simulink model "large_model"  (Simulink 8.0)
settings: Solver=ode45  StartTime=0.0  StopTime=10.0  RelTol=1e-3
(part 8 of 21)

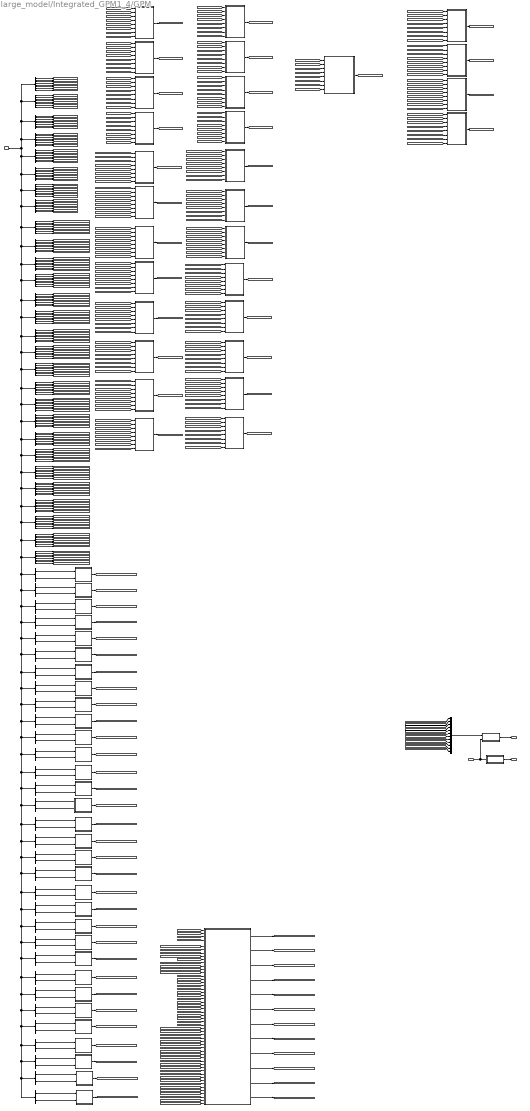
== system large_model/Integrated_GPM1_4/GPM ==
  note: Este componente foi colocado devido a uma limitação do EFTG. Na versão 1.2.1,
      | não é permitido fazer uma conexão direta entre a entrada e a saída.
  From_ES : Inport
  PWR_In : Inport  Port=2
  CAB1_FCC1 : Subsystem
  CAB1_GPM2_fcc_ram_mon_rudder_UPR_dat : BusSelector  OutputSignals=switch2A.switch1A_to_switch.cab1_gpm2_chB.CAB1_GPM2_fcc_ram_mon_rudder_UPR_dat,switch2B.switch1B_to_switch.cab1_gpm2_chB.CAB1_GPM2_fcc_ram_mon_rudder_UPR_dat
  CAB4_GPM2_fcc_ram_mon_AIL_LIB_dat : BusSelector  OutputSignals=switch2A.cab4_gpm2_chA.CAB4_GPM2_fcc_ram_mon_AIL_LIB_dat,switch2B.cab4_gpm2_chB.CAB4_GPM2_fcc_ram_mon_AIL_LIB_dat
  CAB4_GPM2_fcc_ram_mon_AIL_LOB_dat : BusSelector  OutputSignals=switch2A.cab4_gpm2_chA.CAB4_GPM2_fcc_ram_mon_AIL_LOB_dat,switch2B.cab4_gpm2_chB.CAB4_GPM2_fcc_ram_mon_AIL_LOB_dat
  CAB4_GPM2_fcc_ram_mon_AIL_RIB_dat : BusSelector  OutputSignals=switch2A.cab4_gpm2_chA.CAB4_GPM2_fcc_ram_mon_AIL_RIB_dat,switch2B.cab4_gpm2_chB.CAB4_GPM2_fcc_ram_mon_AIL_RIB_dat
  CAB4_GPM2_fcc_ram_mon_AIL_ROB_dat : BusSelector  OutputSignals=switch2A.cab4_gpm2_chA.CAB4_GPM2_fcc_ram_mon_AIL_ROB_dat,switch2B.cab4_gpm2_chB.CAB4_GPM2_fcc_ram_mon_AIL_ROB_dat
  CAB4_GPM2_fcc_ram_mon_ELEV_LIB_dat : BusSelector  OutputSignals=switch2A.cab4_gpm2_chA.CAB4_GPM2_fcc_ram_mon_ELEV_LIB_dat,switch2B.cab4_gpm2_chB.CAB4_GPM2_fcc_ram_mon_ELEV_LIB_dat
  CAB4_GPM2_fcc_ram_mon_ELEV_LOB_dat : BusSelector  OutputSignals=switch2A.cab4_gpm2_chA.CAB4_GPM2_fcc_ram_mon_ELEV_LOB_dat,switch2B.cab4_gpm2_chB.CAB4_GPM2_fcc_ram_mon_ELEV_LOB_dat
  CAB4_GPM2_fcc_ram_mon_ELEV_RIB_dat : BusSelector  OutputSignals=switch2A.cab4_gpm2_chA.CAB4_GPM2_fcc_ram_mon_ELEV_RIB_dat,switch2B.cab4_gpm2_chB.CAB4_GPM2_fcc_ram_mon_ELEV_RIB_dat
  CAB4_GPM2_fcc_ram_mon_ELEV_ROB_dat : BusSelector  OutputSignals=switch2A.cab4_gpm2_chA.CAB4_GPM2_fcc_ram_mon_ELEV_ROB_dat,switch2B.cab4_gpm2_chB.CAB4_GPM2_fcc_ram_mon_ELEV_ROB_dat
  CAB4_GPM2_fcc_ram_mon_rudder_LWR_dat : BusSelector  OutputSignals=switch2A.cab4_gpm2_chA.CAB4_GPM2_fcc_ram_mon_rudder_LWR_dat,switch2B.cab4_gpm2_chB.CAB4_GPM2_fcc_ram_mon_rudder_LWR_dat
  CAB4_GPM2_pitch_roll_cmd : BusSelector  OutputSignals=switch2A.cab4_gpm2_chA.CAB4_GPM2_pitch_roll_cmd,switch2B.cab4_gpm2_chB.CAB4_GPM2_pitch_roll_cmd
  CAB4_GPM2_yaw_cmd : BusSelector  OutputSignals=switch2A.cab4_gpm2_chA.CAB4_GPM2_yaw_cmd,switch2B.cab4_gpm2_chB.CAB4_GPM2_yaw_cmd
  GPM Hardware : Subsystem
  Outputs : BusCreator  Inputs=12
  PWR : Subsystem
  SCP_CAB1_GPM2_fcc_ram_mon_AIL_LIB : Subsystem
  SCP_CAB1_GPM2_fcc_ram_mon_AIL_LOB : Subsystem
  SCP_CAB1_GPM2_fcc_ram_mon_AIL_RIB : Subsystem
  SCP_CAB1_GPM2_fcc_ram_mon_AIL_ROB : Subsystem
  SCP_CAB1_GPM2_fcc_ram_mon_ELEV_LIB : Subsystem
  SCP_CAB1_GPM2_fcc_ram_mon_ELEV_LOB : Subsystem
  SCP_CAB1_GPM2_fcc_ram_mon_ELEV_RIB : Subsystem
  SCP_CAB1_GPM2_fcc_ram_mon_ELEV_ROB : Subsystem
  SCP_CAB1_GPM2_fcc_ram_mon_rudder_LWR : Subsystem
  SCP_CAB1_GPM2_fcc_ram_mon_rudder_UPR : Subsystem
  SCP_CAB1_GPM2_pitch_roll : Subsystem
  SCP_CAB1_GPM2_yaw : Subsystem
  SCP_ail_REU_LIB_LVDT : Subsystem
  SCP_ail_REU_LIB_mon : Subsystem
  SCP_ail_REU_LOB_LVDT : Subsystem
  SCP_ail_REU_LOB_mon : Subsystem
  SCP_ail_REU_RIB_LVDT : Subsystem
  SCP_ail_REU_RIB_mon : Subsystem
  SCP_ail_REU_ROB_LVDT : Subsystem
  SCP_ail_REU_ROB_mon : Subsystem
  SCP_elev_REU_LIB_LVDT : Subsystem
  SCP_elev_REU_LIB_mon_dat : Subsystem
  SCP_elev_REU_LOB_LVDT : Subsystem
  SCP_elev_REU_LOB_mon : Subsystem
  SCP_elev_REU_RIB_LVDT : Subsystem
  SCP_elev_REU_RIB_mon : Subsystem
  SCP_elev_REU_ROB_LVDT : Subsystem
  SCP_elev_REU_ROB_mon : Subsystem
  SCP_rud_REU1_LVDT : Subsystem
  SCP_rud_REU1_MON : Subsystem
  SCP_rud_REU2_LVDT : Subsystem
  SCP_rud_REU2_MON : Subsystem
  Self_checking_pair_ADS1A : Subsystem
  Self_checking_pair_ADS1B : Subsystem
  Self_checking_pair_ADS2A : Subsystem
  Self_checking_pair_ADS2B : Subsystem
  Self_checking_pair_ADS3A : Subsystem
  Self_checking_pair_ADS3B : Subsystem
  Self_checking_pair_ADS4A : Subsystem
  Self_checking_pair_ADS4B : Subsystem
  Self_checking_pair_PedalYaw1 : Subsystem
  Self_checking_pair_PedalYaw2 : Subsystem
  Self_checking_pair_PedalYaw3 : Subsystem
  Self_checking_pair_PedalYaw4 : Subsystem
  Self_checking_pair_SSC1_pitch1 : Subsystem
  Self_checking_pair_SSC1_pitch2 : Subsystem
  Self_checking_pair_SSC1_pitch3 : Subsystem
  Self_checking_pair_SSC1_pitch4 : Subsystem
  Self_checking_pair_SSC1_roll1 : Subsystem
  Self_checking_pair_SSC1_roll2 : Subsystem
  Self_checking_pair_SSC1_roll3 : Subsystem
  Self_checking_pair_SSC1_roll4 : Subsystem
  Self_checking_pair_SSC2_pitch1 : Subsystem
  Self_checking_pair_SSC2_pitch2 : Subsystem
  Self_checking_pair_SSC2_pitch3 : Subsystem
  Self_checking_pair_SSC2_pitch4 : Subsystem
  Self_checking_pair_SSC2_roll1 : Subsystem
  Self_checking_pair_SSC2_roll2 : Subsystem
  Self_checking_pair_SSC2_roll3 : Subsystem
  Self_checking_pair_SSC2_roll4 : Subsystem
  Voter_ADS : Subsystem
  ads_1A : BusSelector  Description=|  OutputSignals=switch2A.cab3_gpm_IO_chA.ads_1A,switch2A.cab4_gpm_IO_chA.ads_1A,switch2A.switch1A_to_switch.cab2_gpm_IO_chB.ads_1A,switch2A.switch1A_to_switch.cab1_gpm_IO_chB.ads_1A,switch2B.cab4_gpm_IO_chB.ads_1A,switch2B.cab3_gpm_IO_chB.ads_1A,switch2B.switch1B_to_switch.cab2_gpm_IO_chB.ads_1A,switch2B.switch1B_to_switch.cab1_gpm_IO_chB.ads_1A
      | When connected to a line which contains bus signals, this bus selector will select as its outputs all the signals which contain this bus selector name.
      | For example, if you name this bus selector as "sidestick_1_pitch_1", all signals containing this string will be selected as its outputs.
  ads_1B : BusSelector  Description=|  OutputSignals=switch2A.cab3_gpm_IO_chA.ads_1B,switch2A.cab4_gpm_IO_chA.ads_1B,switch2A.switch1A_to_switch.cab2_gpm_IO_chB.ads_1B,switch2A.switch1A_to_switch.cab1_gpm_IO_chB.ads_1B,switch2B.cab4_gpm_IO_chB.ads_1B,switch2B.cab3_gpm_IO_chB.ads_1B,switch2B.switch1B_to_switch.cab2_gpm_IO_chB.ads_1B,switch2B.switch1B_to_switch.cab1_gpm_IO_chB.ads_1B
      | When connected to a line which contains bus signals, this bus selector will select as its outputs all the signals which contain this bus selector name.
      | For example, if you name this bus selector as "sidestick_1_pitch_1", all signals containing this string will be selected as its outputs.
  ads_2A : BusSelector  Description=|  OutputSignals=switch2A.cab3_gpm_IO_chA.ads_2A,switch2A.cab4_gpm_IO_chA.ads_2A,switch2A.switch1A_to_switch.cab2_gpm_IO_chB.ads_2A,switch2A.switch1A_to_switch.cab1_gpm_IO_chB.ads_2A,switch2B.cab4_gpm_IO_chB.ads_2A,switch2B.cab3_gpm_IO_chB.ads_2A,switch2B.switch1B_to_switch.cab2_gpm_IO_chB.ads_2A,switch2B.switch1B_to_switch.cab1_gpm_IO_chB.ads_2A
      | When connected to a line which contains bus signals, this bus selector will select as its outputs all the signals which contain this bus selector name.
      | For example, if you name this bus selector as "sidestick_1_pitch_1", all signals containing this string will be selected as its outputs.
  ads_2B : BusSelector  Description=|  OutputSignals=switch2A.cab3_gpm_IO_chA.ads_2B,switch2A.cab4_gpm_IO_chA.ads_2B,switch2A.switch1A_to_switch.cab2_gpm_IO_chB.ads_2B,switch2A.switch1A_to_switch.cab1_gpm_IO_chB.ads_2B,switch2B.cab4_gpm_IO_chB.ads_2B,switch2B.cab3_gpm_IO_chB.ads_2B,switch2B.switch1B_to_switch.cab2_gpm_IO_chB.ads_2B,switch2B.switch1B_to_switch.cab1_gpm_IO_chB.ads_2B
      | When connected to a line which contains bus signals, this bus selector will select as its outputs all the signals which contain this bus selector name.
      | For example, if you name this bus selector as "sidestick_1_pitch_1", all signals containing this string will be selected as its outputs.
  ads_3A : BusSelector  Description=|  OutputSignals=switch2A.cab3_gpm_IO_chA.ads_3A,switch2A.cab4_gpm_IO_chA.ads_3A,switch2A.switch1A_to_switch.cab2_gpm_IO_chB.ads_3A,switch2A.switch1A_to_switch.cab1_gpm_IO_chB.ads_3A,switch2B.cab4_gpm_IO_chB.ads_3A,switch2B.cab3_gpm_IO_chB.ads_3A,switch2B.switch1B_to_switch.cab2_gpm_IO_chB.ads_3A,switch2B.switch1B_to_switch.cab1_gpm_IO_chB.ads_3A
      | When connected to a line which contains bus signals, this bus selector will select as its outputs all the signals which contain this bus selector name.
      | For example, if you name this bus selector as "sidestick_1_pitch_1", all signals containing this string will be selected as its outputs.
  ads_3B : BusSelector  Description=|  OutputSignals=switch2A.cab3_gpm_IO_chA.ads_3B,switch2A.cab4_gpm_IO_chA.ads_3B,switch2A.switch1A_to_switch.cab2_gpm_IO_chB.ads_3B,switch2A.switch1A_to_switch.cab1_gpm_IO_chB.ads_3B,switch2B.cab4_gpm_IO_chB.ads_3B,switch2B.cab3_gpm_IO_chB.ads_3B,switch2B.switch1B_to_switch.cab2_gpm_IO_chB.ads_3B,switch2B.switch1B_to_switch.cab1_gpm_IO_chB.ads_3B
      | When connected to a line which contains bus signals, this bus selector will select as its outputs all the signals which contain this bus selector name.
      | For example, if you name this bus selector as "sidestick_1_pitch_1", all signals containing this string will be selected as its outputs.
  ads_4A : BusSelector  Description=|  OutputSignals=switch2A.cab3_gpm_IO_chA.ads_4A,switch2A.cab4_gpm_IO_chA.ads_4A,switch2A.switch1A_to_switch.cab2_gpm_IO_chB.ads_4A,switch2A.switch1A_to_switch.cab1_gpm_IO_chB.ads_4A,switch2B.cab4_gpm_IO_chB.ads_4A,switch2B.cab3_gpm_IO_chB.ads_4A,switch2B.switch1B_to_switch.cab2_gpm_IO_chB.ads_4A,switch2B.switch1B_to_switch.cab1_gpm_IO_chB.ads_4A
      | When connected to a line which contains bus signals, this bus selector will select as its outputs all the signals which contain this bus selector name.
      | For example, if you name this bus selector as "sidestick_1_pitch_1", all signals containing this string will be selected as its outputs.
  ads_4B : BusSelector  Description=|  OutputSignals=switch2A.cab3_gpm_IO_chA.ads_4B,switch2A.cab4_gpm_IO_chA.ads_4B,switch2A.switch1A_to_switch.cab2_gpm_IO_chB.ads_4B,switch2A.switch1A_to_switch.cab1_gpm_IO_chB.ads_4B,switch2B.cab4_gpm_IO_chB.ads_4B,switch2B.cab3_gpm_IO_chB.ads_4B,switch2B.switch1B_to_switch.cab2_gpm_IO_chB.ads_4B,switch2B.switch1B_to_switch.cab1_gpm_IO_chB.ads_4B
      | When connected to a line which contains bus signals, this bus selector will select as its outputs all the signals which contain this bus selector name.
      | For example, if you name this bus selector as "sidestick_1_pitch_1", all signals containing this string will be selected as its outputs.
  ail_REU_LIB_LVDT_dat : BusSelector  OutputSignals=switch2A.REU_to_Switch2A.ail_REU_LIB_LVDT_dat,switch2B.REU_to_Switch2B.ail_REU_LIB_LVDT_dat
  ail_REU_LIB_mon_dat : BusSelector  OutputSignals=switch2A.REU_to_Switch2A.ail_REU_LIB_mon_dat,switch2B.REU_to_Switch2B.ail_REU_LIB_mon_dat
  ail_REU_LOB_LVDT_dat : BusSelector  OutputSignals=switch2A.switch1A_to_switch.REU_to_Switch1A.ail_REU_LOB_LVDT_dat,switch2B.switch1B_to_switch.REU_to_Switch1B.ail_REU_LOB_LVDT_dat
  ail_REU_LOB_mon_dat : BusSelector  OutputSignals=switch2A.switch1A_to_switch.REU_to_Switch1A.ail_REU_LOB_mon_dat,switch2B.switch1B_to_switch.REU_to_Switch1B.ail_REU_LOB_mon_dat
  ail_REU_RIB_LVDT_dat : BusSelector  OutputSignals=switch2A.REU_to_Switch2A.ail_REU_RIB_LVDT_dat,switch2B.REU_to_Switch2B.ail_REU_RIB_LVDT_dat
  ail_REU_RIB_mon_dat : BusSelector  OutputSignals=switch2A.REU_to_Switch2A.ail_REU_RIB_mon_dat,switch2B.REU_to_Switch2B.ail_REU_RIB_mon_dat
  ail_REU_ROB_LVDT_dat : BusSelector  OutputSignals=switch2A.switch1A_to_switch.REU_to_Switch1A.ail_REU_ROB_LVDT_dat,switch2B.switch1B_to_switch.REU_to_Switch1B.ail_REU_ROB_LVDT_dat
  ail_REU_ROB_mon_dat : BusSelector  OutputSignals=switch2A.switch1A_to_switch.REU_to_Switch1A.ail_REU_ROB_mon_dat,switch2B.switch1B_to_switch.REU_to_Switch1B.ail_REU_ROB_mon_dat
  elev_REU_LIB_LVDT_dat : BusSelector  OutputSignals=switch2A.REU_to_Switch2A.elev_REU_LIB_LVDT_dat,switch2B.REU_to_Switch2B.elev_REU_LIB_LVDT_dat
  elev_REU_LIB_mon_dat : BusSelector  OutputSignals=switch2A.REU_to_Switch2A.elev_REU_LIB_mon_dat,switch2B.REU_to_Switch2B.elev_REU_LIB_mon_dat
  elev_REU_LOB_LVDT_dat : BusSelector  OutputSignals=switch2A.switch1A_to_switch.REU_to_Switch1A.elev_REU_LOB_LVDT_dat,switch2B.switch1B_to_switch.REU_to_Switch1B.elev_REU_LOB_LVDT_dat
  elev_REU_LOB_mon_dat : BusSelector  OutputSignals=switch2A.switch1A_to_switch.REU_to_Switch1A.elev_REU_LOB_mon_dat,switch2B.switch1B_to_switch.REU_to_Switch1B.elev_REU_LOB_mon_dat
  elev_REU_RIB_LVDT_dat : BusSelector  OutputSignals=switch2A.REU_to_Switch2A.elev_REU_RIB_LVDT_dat,switch2B.REU_to_Switch2B.elev_REU_RIB_LVDT_dat
  elev_REU_RIB_mon_dat : BusSelector  OutputSignals=switch2A.REU_to_Switch2A.elev_REU_RIB_mon_dat,switch2B.REU_to_Switch2B.elev_REU_RIB_mon_dat
  elev_REU_ROB_LVDT_dat : BusSelector  OutputSignals=switch2A.switch1A_to_switch.REU_to_Switch1A.elev_REU_ROB_LVDT_dat,switch2B.switch1B_to_switch.REU_to_Switch1B.elev_REU_ROB_LVDT_dat
  elev_REU_ROB_mon_dat : BusSelector  OutputSignals=switch2A.switch1A_to_switch.REU_to_Switch1A.elev_REU_ROB_mon_dat,switch2B.switch1B_to_switch.REU_to_Switch1B.elev_REU_ROB_mon_dat
  pedal_yaw_1 : BusSelector  Description=|  OutputSignals=switch2A.cab3_gpm_IO_chA.rdc_4002_1.pedal_yaw_1,switch2A.cab4_gpm_IO_chA.rdc_4002_1.pedal_yaw_1,switch2A.switch1A_to_switch.cab2_gpm_IO_chB.rdc_4002_1.pedal_yaw_1,switch2A.switch1A_to_switch.cab1_gpm_IO_chB.rdc_4002_1.pedal_yaw_1,switch2B.cab4_gpm_IO_chB.rdc_4002_1.pedal_yaw_1,switch2B.cab3_gpm_IO_chB.rdc_4002_1.pedal_yaw_1,switch2B.switch1B_to_switch.cab2_gpm_IO_chB.rdc_4002_1.pedal_yaw_1,switch2B.switch1B_to_switch.cab1_gpm_IO_chB.rdc_4002_1.pedal_yaw_1
      | When connected to a line which contains bus signals, this bus selector will select as its outputs all the signals which contain this bus selector name.
      | For example, if you name this bus selector as "sidestick_1_pitch_1", all signals containing this string will be selected as its outputs.
  pedal_yaw_2 : BusSelector  Description=|  OutputSignals=switch2A.cab3_gpm_IO_chA.rdc_4002_2.pedal_yaw_2,switch2A.cab4_gpm_IO_chA.rdc_4002_2.pedal_yaw_2,switch2A.switch1A_to_switch.cab2_gpm_IO_chB.rdc_4002_2.pedal_yaw_2,switch2A.switch1A_to_switch.cab1_gpm_IO_chB.rdc_4002_2.pedal_yaw_2,switch2B.cab4_gpm_IO_chB.rdc_4002_2.pedal_yaw_2,switch2B.cab3_gpm_IO_chB.rdc_4002_2.pedal_yaw_2,switch2B.switch1B_to_switch.cab2_gpm_IO_chB.rdc_4002_2.pedal_yaw_2,switch2B.switch1B_to_switch.cab1_gpm_IO_chB.rdc_4002_2.pedal_yaw_2
      | When connected to a line which contains bus signals, this bus selector will select as its outputs all the signals which contain this bus selector name.
      | For example, if you name this bus selector as "sidestick_1_pitch_1", all signals containing this string will be selected as its outputs.
  pedal_yaw_3 : BusSelector  Description=|  OutputSignals=switch2A.cab3_gpm_IO_chA.rdc_4002_3.pedal_yaw_3,switch2A.cab4_gpm_IO_chA.rdc_4002_3.pedal_yaw_3,switch2A.switch1A_to_switch.cab2_gpm_IO_chB.rdc_4002_3.pedal_yaw_3,switch2A.switch1A_to_switch.cab1_gpm_IO_chB.rdc_4002_3.pedal_yaw_3,switch2B.cab4_gpm_IO_chB.rdc_4002_3.pedal_yaw_3,switch2B.cab3_gpm_IO_chB.rdc_4002_3.pedal_yaw_3,switch2B.switch1B_to_switch.cab2_gpm_IO_chB.rdc_4002_3.pedal_yaw_3,switch2B.switch1B_to_switch.cab1_gpm_IO_chB.rdc_4002_3.pedal_yaw_3
      | When connected to a line which contains bus signals, this bus selector will select as its outputs all the signals which contain this bus selector name.
      | For example, if you name this bus selector as "sidestick_1_pitch_1", all signals containing this string will be selected as its outputs.
  pedal_yaw_4 : BusSelector  Description=|  OutputSignals=switch2A.cab3_gpm_IO_chA.rdc_4002_4.pedal_yaw_4,switch2A.cab4_gpm_IO_chA.rdc_4002_4.pedal_yaw_4,switch2A.switch1A_to_switch.cab2_gpm_IO_chB.rdc_4002_4.pedal_yaw_4,switch2A.switch1A_to_switch.cab1_gpm_IO_chB.rdc_4002_4.pedal_yaw_4,switch2B.cab4_gpm_IO_chB.rdc_4002_4.pedal_yaw_4,switch2B.cab3_gpm_IO_chB.rdc_4002_4.pedal_yaw_4,switch2B.switch1B_to_switch.cab2_gpm_IO_chB.rdc_4002_4.pedal_yaw_4,switch2B.switch1B_to_switch.cab1_gpm_IO_chB.rdc_4002_4.pedal_yaw_4
      | When connected to a line which contains bus signals, this bus selector will select as its outputs all the signals which contain this bus selector name.
      | For example, if you name this bus selector as "sidestick_1_pitch_1", all signals containing this string will be selected as its outputs.
  rud_REU1_LVDT_dat : BusSelector  OutputSignals=switch2A.REU_to_Switch2A.rud_REU1_LVDT_dat,switch2B.REU_to_Switch2B.rud_REU1_LVDT_dat
  rud_REU1_MON_dat : BusSelector  OutputSignals=switch2A.REU_to_Switch2A.rud_REU1_MON_dat,switch2B.REU_to_Switch2B.rud_REU1_MON_dat
  rud_REU2_LVDT_dat : BusSelector  OutputSignals=switch2A.switch1A_to_switch.REU_to_Switch1A.rud_REU2_LVDT_dat,switch2B.switch1B_to_switch.REU_to_Switch1B.rud_REU2_LVDT_dat
  rud_REU2_MON_dat : BusSelector  OutputSignals=switch2A.switch1A_to_switch.REU_to_Switch1A.rud_REU2_MON_dat,switch2B.switch1B_to_switch.REU_to_Switch1B.rud_REU2_MON_dat
  sidestick1_pitch_1 : BusSelector  OutputSignals=switch2A.cab3_gpm_IO_chA.rdc_4002_1.sidesticks.sidestick1_pitch_1,switch2A.cab4_gpm_IO_chA.rdc_4002_1.sidesticks.sidestick1_pitch_1,switch2A.switch1A_to_switch.cab2_gpm_IO_chB.rdc_4002_1.sidesticks.sidestick1_pitch_1,switch2A.switch1A_to_switch.cab1_gpm_IO_chB.rdc_4002_1.sidesticks.sidestick1_pitch_1,switch2B.cab4_gpm_IO_chB.rdc_4002_1.sidesticks.sidestick1_pitch_1,switch2B.cab3_gpm_IO_chB.rdc_4002_1.sidesticks.sidestick1_pitch_1,switch2B.switch1B_to_switch.cab2_gpm_IO_chB.rdc_4002_1.sidesticks.sidestick1_pitch_1,switch2B.switch1B_to_switch.cab1_gpm_IO_chB.rdc_4002_1.sidesticks.sidestick1_pitch_1
  sidestick1_pitch_2 : BusSelector  OutputSignals=switch2A.cab3_gpm_IO_chA.rdc_4002_2.sidesticks.sidestick1_pitch_2,switch2A.cab4_gpm_IO_chA.rdc_4002_2.sidesticks.sidestick1_pitch_2,switch2A.switch1A_to_switch.cab2_gpm_IO_chB.rdc_4002_2.sidesticks.sidestick1_pitch_2,switch2A.switch1A_to_switch.cab1_gpm_IO_chB.rdc_4002_2.sidesticks.sidestick1_pitch_2,switch2B.cab4_gpm_IO_chB.rdc_4002_2.sidesticks.sidestick1_pitch_2,switch2B.cab3_gpm_IO_chB.rdc_4002_2.sidesticks.sidestick1_pitch_2,switch2B.switch1B_to_switch.cab2_gpm_IO_chB.rdc_4002_2.sidesticks.sidestick1_pitch_2,switch2B.switch1B_to_switch.cab1_gpm_IO_chB.rdc_4002_2.sidesticks.sidestick1_pitch_2
  sidestick1_pitch_3 : BusSelector  OutputSignals=switch2A.cab3_gpm_IO_chA.rdc_4002_3.sidesticks.sidestick1_pitch_3,switch2A.cab4_gpm_IO_chA.rdc_4002_3.sidesticks.sidestick1_pitch_3,switch2A.switch1A_to_switch.cab2_gpm_IO_chB.rdc_4002_3.sidesticks.sidestick1_pitch_3,switch2A.switch1A_to_switch.cab1_gpm_IO_chB.rdc_4002_3.sidesticks.sidestick1_pitch_3,switch2B.cab4_gpm_IO_chB.rdc_4002_3.sidesticks.sidestick1_pitch_3,switch2B.cab3_gpm_IO_chB.rdc_4002_3.sidesticks.sidestick1_pitch_3,switch2B.switch1B_to_switch.cab2_gpm_IO_chB.rdc_4002_3.sidesticks.sidestick1_pitch_3,switch2B.switch1B_to_switch.cab1_gpm_IO_chB.rdc_4002_3.sidesticks.sidestick1_pitch_3
  sidestick1_pitch_4 : BusSelector  OutputSignals=switch2A.cab3_gpm_IO_chA.rdc_4002_4.sidesticks.sidestick1_pitch_4,switch2A.cab4_gpm_IO_chA.rdc_4002_4.sidesticks.sidestick1_pitch_4,switch2A.switch1A_to_switch.cab2_gpm_IO_chB.rdc_4002_4.sidesticks.sidestick1_pitch_4,switch2A.switch1A_to_switch.cab1_gpm_IO_chB.rdc_4002_4.sidesticks.sidestick1_pitch_4,switch2B.cab4_gpm_IO_chB.rdc_4002_4.sidesticks.sidestick1_pitch_4,switch2B.cab3_gpm_IO_chB.rdc_4002_4.sidesticks.sidestick1_pitch_4,switch2B.switch1B_to_switch.cab2_gpm_IO_chB.rdc_4002_4.sidesticks.sidestick1_pitch_4,switch2B.switch1B_to_switch.cab1_gpm_IO_chB.rdc_4002_4.sidesticks.sidestick1_pitch_4
  sidestick1_roll_1 : BusSelector  OutputSignals=switch2A.cab3_gpm_IO_chA.rdc_4002_1.sidesticks.sidestick1_roll_1,switch2A.cab4_gpm_IO_chA.rdc_4002_1.sidesticks.sidestick1_roll_1,switch2A.switch1A_to_switch.cab2_gpm_IO_chB.rdc_4002_1.sidesticks.sidestick1_roll_1,switch2A.switch1A_to_switch.cab1_gpm_IO_chB.rdc_4002_1.sidesticks.sidestick1_roll_1,switch2B.cab4_gpm_IO_chB.rdc_4002_1.sidesticks.sidestick1_roll_1,switch2B.cab3_gpm_IO_chB.rdc_4002_1.sidesticks.sidestick1_roll_1,switch2B.switch1B_to_switch.cab2_gpm_IO_chB.rdc_4002_1.sidesticks.sidestick1_roll_1,switch2B.switch1B_to_switch.cab1_gpm_IO_chB.rdc_4002_1.sidesticks.sidestick1_roll_1
  sidestick1_roll_2 : BusSelector  OutputSignals=switch2A.cab3_gpm_IO_chA.rdc_4002_2.sidesticks.sidestick1_roll_2,switch2A.cab4_gpm_IO_chA.rdc_4002_2.sidesticks.sidestick1_roll_2,switch2A.switch1A_to_switch.cab2_gpm_IO_chB.rdc_4002_2.sidesticks.sidestick1_roll_2,switch2A.switch1A_to_switch.cab1_gpm_IO_chB.rdc_4002_2.sidesticks.sidestick1_roll_2,switch2B.cab4_gpm_IO_chB.rdc_4002_2.sidesticks.sidestick1_roll_2,switch2B.cab3_gpm_IO_chB.rdc_4002_2.sidesticks.sidestick1_roll_2,switch2B.switch1B_to_switch.cab2_gpm_IO_chB.rdc_4002_2.sidesticks.sidestick1_roll_2,switch2B.switch1B_to_switch.cab1_gpm_IO_chB.rdc_4002_2.sidesticks.sidestick1_roll_2
  sidestick1_roll_3 : BusSelector  OutputSignals=switch2A.cab3_gpm_IO_chA.rdc_4002_3.sidesticks.sidestick1_roll_3,switch2A.cab4_gpm_IO_chA.rdc_4002_3.sidesticks.sidestick1_roll_3,switch2A.switch1A_to_switch.cab2_gpm_IO_chB.rdc_4002_3.sidesticks.sidestick1_roll_3,switch2A.switch1A_to_switch.cab1_gpm_IO_chB.rdc_4002_3.sidesticks.sidestick1_roll_3,switch2B.cab4_gpm_IO_chB.rdc_4002_3.sidesticks.sidestick1_roll_3,switch2B.cab3_gpm_IO_chB.rdc_4002_3.sidesticks.sidestick1_roll_3,switch2B.switch1B_to_switch.cab2_gpm_IO_chB.rdc_4002_3.sidesticks.sidestick1_roll_3,switch2B.switch1B_to_switch.cab1_gpm_IO_chB.rdc_4002_3.sidesticks.sidestick1_roll_3
  sidestick1_roll_4 : BusSelector  OutputSignals=switch2A.cab3_gpm_IO_chA.rdc_4002_4.sidesticks.sidestick1_roll_4,switch2A.cab4_gpm_IO_chA.rdc_4002_4.sidesticks.sidestick1_roll_4,switch2A.switch1A_to_switch.cab2_gpm_IO_chB.rdc_4002_4.sidesticks.sidestick1_roll_4,switch2A.switch1A_to_switch.cab1_gpm_IO_chB.rdc_4002_4.sidesticks.sidestick1_roll_4,switch2B.cab4_gpm_IO_chB.rdc_4002_4.sidesticks.sidestick1_roll_4,switch2B.cab3_gpm_IO_chB.rdc_4002_4.sidesticks.sidestick1_roll_4,switch2B.switch1B_to_switch.cab2_gpm_IO_chB.rdc_4002_4.sidesticks.sidestick1_roll_4,switch2B.switch1B_to_switch.cab1_gpm_IO_chB.rdc_4002_4.sidesticks.sidestick1_roll_4
  sidestick2_pitch_1 : BusSelector  OutputSignals=switch2A.cab3_gpm_IO_chA.rdc_4002_1.sidesticks.sidestick2_pitch_1,switch2A.cab4_gpm_IO_chA.rdc_4002_1.sidesticks.sidestick2_pitch_1,switch2A.switch1A_to_switch.cab2_gpm_IO_chB.rdc_4002_1.sidesticks.sidestick2_pitch_1,switch2A.switch1A_to_switch.cab1_gpm_IO_chB.rdc_4002_1.sidesticks.sidestick2_pitch_1,switch2B.cab4_gpm_IO_chB.rdc_4002_1.sidesticks.sidestick2_pitch_1,switch2B.cab3_gpm_IO_chB.rdc_4002_1.sidesticks.sidestick2_pitch_1,switch2B.switch1B_to_switch.cab2_gpm_IO_chB.rdc_4002_1.sidesticks.sidestick2_pitch_1,switch2B.switch1B_to_switch.cab1_gpm_IO_chB.rdc_4002_1.sidesticks.sidestick2_pitch_1
  sidestick2_pitch_2 : BusSelector  OutputSignals=switch2A.cab3_gpm_IO_chA.rdc_4002_2.sidesticks.sidestick2_pitch_2,switch2A.cab4_gpm_IO_chA.rdc_4002_2.sidesticks.sidestick2_pitch_2,switch2A.switch1A_to_switch.cab2_gpm_IO_chB.rdc_4002_2.sidesticks.sidestick2_pitch_2,switch2A.switch1A_to_switch.cab1_gpm_IO_chB.rdc_4002_2.sidesticks.sidestick2_pitch_2,switch2B.cab4_gpm_IO_chB.rdc_4002_2.sidesticks.sidestick2_pitch_2,switch2B.cab3_gpm_IO_chB.rdc_4002_2.sidesticks.sidestick2_pitch_2,switch2B.switch1B_to_switch.cab2_gpm_IO_chB.rdc_4002_2.sidesticks.sidestick2_pitch_2,switch2B.switch1B_to_switch.cab1_gpm_IO_chB.rdc_4002_2.sidesticks.sidestick2_pitch_2
  sidestick2_pitch_3 : BusSelector  OutputSignals=switch2A.cab3_gpm_IO_chA.rdc_4002_3.sidesticks.sidestick2_pitch_3,switch2A.cab4_gpm_IO_chA.rdc_4002_3.sidesticks.sidestick2_pitch_3,switch2A.switch1A_to_switch.cab2_gpm_IO_chB.rdc_4002_3.sidesticks.sidestick2_pitch_3,switch2A.switch1A_to_switch.cab1_gpm_IO_chB.rdc_4002_3.sidesticks.sidestick2_pitch_3,switch2B.cab4_gpm_IO_chB.rdc_4002_3.sidesticks.sidestick2_pitch_3,switch2B.cab3_gpm_IO_chB.rdc_4002_3.sidesticks.sidestick2_pitch_3,switch2B.switch1B_to_switch.cab2_gpm_IO_chB.rdc_4002_3.sidesticks.sidestick2_pitch_3,switch2B.switch1B_to_switch.cab1_gpm_IO_chB.rdc_4002_3.sidesticks.sidestick2_pitch_3
  sidestick2_pitch_4 : BusSelector  OutputSignals=switch2A.cab3_gpm_IO_chA.rdc_4002_4.sidesticks.sidestick2_pitch_4,switch2A.cab4_gpm_IO_chA.rdc_4002_4.sidesticks.sidestick2_pitch_4,switch2A.switch1A_to_switch.cab2_gpm_IO_chB.rdc_4002_4.sidesticks.sidestick2_pitch_4,switch2A.switch1A_to_switch.cab1_gpm_IO_chB.rdc_4002_4.sidesticks.sidestick2_pitch_4,switch2B.cab4_gpm_IO_chB.rdc_4002_4.sidesticks.sidestick2_pitch_4,switch2B.cab3_gpm_IO_chB.rdc_4002_4.sidesticks.sidestick2_pitch_4,switch2B.switch1B_to_switch.cab2_gpm_IO_chB.rdc_4002_4.sidesticks.sidestick2_pitch_4,switch2B.switch1B_to_switch.cab1_gpm_IO_chB.rdc_4002_4.sidesticks.sidestick2_pitch_4
  sidestick2_roll_1 : BusSelector  OutputSignals=switch2A.cab3_gpm_IO_chA.rdc_4002_1.sidesticks.sidestick2_roll_1,switch2A.cab4_gpm_IO_chA.rdc_4002_1.sidesticks.sidestick2_roll_1,switch2A.switch1A_to_switch.cab2_gpm_IO_chB.rdc_4002_1.sidesticks.sidestick2_roll_1,switch2A.switch1A_to_switch.cab1_gpm_IO_chB.rdc_4002_1.sidesticks.sidestick2_roll_1,switch2B.cab4_gpm_IO_chB.rdc_4002_1.sidesticks.sidestick2_roll_1,switch2B.cab3_gpm_IO_chB.rdc_4002_1.sidesticks.sidestick2_roll_1,switch2B.switch1B_to_switch.cab2_gpm_IO_chB.rdc_4002_1.sidesticks.sidestick2_roll_1,switch2B.switch1B_to_switch.cab1_gpm_IO_chB.rdc_4002_1.sidesticks.sidestick2_roll_1
  sidestick2_roll_2 : BusSelector  OutputSignals=switch2A.cab3_gpm_IO_chA.rdc_4002_2.sidesticks.sidestick2_roll_2,switch2A.cab4_gpm_IO_chA.rdc_4002_2.sidesticks.sidestick2_roll_2,switch2A.switch1A_to_switch.cab2_gpm_IO_chB.rdc_4002_2.sidesticks.sidestick2_roll_2,switch2A.switch1A_to_switch.cab1_gpm_IO_chB.rdc_4002_2.sidesticks.sidestick2_roll_2,switch2B.cab4_gpm_IO_chB.rdc_4002_2.sidesticks.sidestick2_roll_2,switch2B.cab3_gpm_IO_chB.rdc_4002_2.sidesticks.sidestick2_roll_2,switch2B.switch1B_to_switch.cab2_gpm_IO_chB.rdc_4002_2.sidesticks.sidestick2_roll_2,switch2B.switch1B_to_switch.cab1_gpm_IO_chB.rdc_4002_2.sidesticks.sidestick2_roll_2
  sidestick2_roll_3 : BusSelector  OutputSignals=switch2A.cab3_gpm_IO_chA.rdc_4002_3.sidesticks.sidestick2_roll_3,switch2A.cab4_gpm_IO_chA.rdc_4002_3.sidesticks.sidestick2_roll_3,switch2A.switch1A_to_switch.cab2_gpm_IO_chB.rdc_4002_3.sidesticks.sidestick2_roll_3,switch2A.switch1A_to_switch.cab1_gpm_IO_chB.rdc_4002_3.sidesticks.sidestick2_roll_3,switch2B.cab4_gpm_IO_chB.rdc_4002_3.sidesticks.sidestick2_roll_3,switch2B.cab3_gpm_IO_chB.rdc_4002_3.sidesticks.sidestick2_roll_3,switch2B.switch1B_to_switch.cab2_gpm_IO_chB.rdc_4002_3.sidesticks.sidestick2_roll_3,switch2B.switch1B_to_switch.cab1_gpm_IO_chB.rdc_4002_3.sidesticks.sidestick2_roll_3
  sidestick2_roll_4 : BusSelector  OutputSignals=switch2A.cab3_gpm_IO_chA.rdc_4002_4.sidesticks.sidestick2_roll_4,switch2A.cab4_gpm_IO_chA.rdc_4002_4.sidesticks.sidestick2_roll_4,switch2A.switch1A_to_switch.cab2_gpm_IO_chB.rdc_4002_4.sidesticks.sidestick2_roll_4,switch2A.switch1A_to_switch.cab1_gpm_IO_chB.rdc_4002_4.sidesticks.sidestick2_roll_4,switch2B.cab4_gpm_IO_chB.rdc_4002_4.sidesticks.sidestick2_roll_4,switch2B.cab3_gpm_IO_chB.rdc_4002_4.sidesticks.sidestick2_roll_4,switch2B.switch1B_to_switch.cab2_gpm_IO_chB.rdc_4002_4.sidesticks.sidestick2_roll_4,switch2B.switch1B_to_switch.cab1_gpm_IO_chB.rdc_4002_4.sidesticks.sidestick2_roll_4
  To_ES : Outport
  PWR_Out : Outport  Port=2
  wires:
  [SCP_CAB1_GPM2_yaw_1] -> CAB1_FCC1/6(yaw_GPM2_cmd)
  SCP_CAB1_GPM2_yaw/1(Out) -> [SCP_CAB1_GPM2_yaw_1]
  [SCP_CAB1_GPM2_fcc_ram_mon_rudder_LWR_1] -> CAB1_FCC1/45(fcc_ram_mon_rudder_LWR_GPM2_dat)
  SCP_CAB1_GPM2_fcc_ram_mon_rudder_LWR/1(Out) -> [SCP_CAB1_GPM2_fcc_ram_mon_rudder_LWR_1]
  [SCP_CAB1_GPM2_fcc_ram_mon_AIL_ROB_1] -> CAB1_FCC1/50(fcc_ram_mon_AIL_ROB_GPM2_dat)
  SCP_CAB1_GPM2_fcc_ram_mon_AIL_ROB/1(Out) -> [SCP_CAB1_GPM2_fcc_ram_mon_AIL_ROB_1]
  [SCP_CAB1_GPM2_fcc_ram_mon_AIL_RIB_1] -> CAB1_FCC1/49(fcc_ram_mon_AIL_RIB_GPM2_dat)
  SCP_CAB1_GPM2_fcc_ram_mon_AIL_RIB/1(Out) -> [SCP_CAB1_GPM2_fcc_ram_mon_AIL_RIB_1]
  [SCP_CAB1_GPM2_fcc_ram_mon_AIL_LOB_1] -> CAB1_FCC1/48(fcc_ram_mon_AIL_LOB_GPM2_dat)
  SCP_CAB1_GPM2_fcc_ram_mon_AIL_LOB/1(Out) -> [SCP_CAB1_GPM2_fcc_ram_mon_AIL_LOB_1]
  [SCP_CAB1_GPM2_fcc_ram_mon_AIL_LIB_1] -> CAB1_FCC1/47(fcc_ram_mon_AIL_LIB_GPM2_dat)
  SCP_CAB1_GPM2_fcc_ram_mon_AIL_LIB/1(Out) -> [SCP_CAB1_GPM2_fcc_ram_mon_AIL_LIB_1]
  [SCP_CAB1_GPM2_fcc_ram_mon_rudder_UPR_1] -> CAB1_FCC1/46(fcc_ram_mon_rudder_UPR_GPM2_dat)
  SCP_CAB1_GPM2_fcc_ram_mon_rudder_UPR/1(Out) -> [SCP_CAB1_GPM2_fcc_ram_mon_rudder_UPR_1]
  [SCP_CAB1_GPM2_pitch_roll_1] -> CAB1_FCC1/31(pitch_roll_GPM2_cmd)
  SCP_CAB1_GPM2_pitch_roll/1(Out) -> [SCP_CAB1_GPM2_pitch_roll_1]
  [SCP_CAB1_GPM2_fcc_ram_mon_ELEV_ROB_1] -> CAB1_FCC1/54(fcc_ram_mon_ELEV_ROB_GPM2_dat)
  SCP_CAB1_GPM2_fcc_ram_mon_ELEV_ROB/1(Out) -> [SCP_CAB1_GPM2_fcc_ram_mon_ELEV_ROB_1]
  [SCP_CAB1_GPM2_fcc_ram_mon_ELEV_RIB_1] -> CAB1_FCC1/53(fcc_ram_mon_ELEV_RIB_GPM2_da)
  SCP_CAB1_GPM2_fcc_ram_mon_ELEV_RIB/1(Out) -> [SCP_CAB1_GPM2_fcc_ram_mon_ELEV_RIB_1]
  [SCP_CAB1_GPM2_fcc_ram_mon_ELEV_LOB_1] -> CAB1_FCC1/52(fcc_ram_mon_ELEV_LOB_GPM2_dat)
  SCP_CAB1_GPM2_fcc_ram_mon_ELEV_LOB/1(Out) -> [SCP_CAB1_GPM2_fcc_ram_mon_ELEV_LOB_1]
  [SCP_CAB1_GPM2_fcc_ram_mon_ELEV_LIB_1] -> CAB1_FCC1/51(fcc_ram_mon_ELEV_LIB_GPM2_dat)
  SCP_CAB1_GPM2_fcc_ram_mon_ELEV_LIB/1(Out) -> [SCP_CAB1_GPM2_fcc_ram_mon_ELEV_LIB_1]
  [SCP_elev_REU_ROB_mon_1] -> CAB1_FCC1/44(REU_ELEV_mon_ROB_dat)
  SCP_elev_REU_ROB_mon/1(Out) -> [SCP_elev_REU_ROB_mon_1]
  [SCP_ail_REU_LIB_mon_1] -> CAB1_FCC1/8(REU_mon_AIL_LIB_dat)
  SCP_ail_REU_LIB_mon/1(Out) -> [SCP_ail_REU_LIB_mon_1]
  [SCP_ail_REU_LOB_LVDT_1] -> CAB1_FCC1/9(REU_LVDT_AIL_LOB_dat)
  SCP_ail_REU_LOB_LVDT/1(Out) -> [SCP_ail_REU_LOB_LVDT_1]
  [SCP_ail_REU_RIB_LVDT_1] -> CAB1_FCC1/11(REU_AIL_LVDT_RIB_dat)
  SCP_ail_REU_RIB_LVDT/1(Out) -> [SCP_ail_REU_RIB_LVDT_1]
  [SCP_ail_REU_RIB_mon_1] -> CAB1_FCC1/12(REU_AIL_mon_RIB_dat)
  SCP_ail_REU_RIB_mon/1(Out) -> [SCP_ail_REU_RIB_mon_1]
  [SCP_ail_REU_ROB_LVDT_1] -> CAB1_FCC1/13(REU_AIL_LVDT_ROB_dat)
  SCP_ail_REU_ROB_LVDT/1(Out) -> [SCP_ail_REU_ROB_LVDT_1]
  [SCP_ail_REU_ROB_mon_1] -> CAB1_FCC1/14(REU_AIL_mon_ROB_dat)
  SCP_ail_REU_ROB_mon/1(Out) -> [SCP_ail_REU_ROB_mon_1]
  [SCP_elev_REU_LIB_LVDT_1] -> CAB1_FCC1/32(REU_ELEV_LVDT_LIB_dat)
  SCP_elev_REU_LIB_LVDT/1(Out) -> [SCP_elev_REU_LIB_LVDT_1]
  [SCP_elev_REU_LIB_mon_dat_1] -> CAB1_FCC1/33(REU_ELEV_mon_LIB_dat)
  SCP_elev_REU_LIB_mon_dat/1(Out) -> [SCP_elev_REU_LIB_mon_dat_1]
  [SCP_elev_REU_LOB_LVDT_1] -> CAB1_FCC1/34(REU_ELEV_LVDT_LOB_dat)
  SCP_elev_REU_LOB_LVDT/1(Out) -> [SCP_elev_REU_LOB_LVDT_1]
  [SCP_elev_REU_LOB_mon_1] -> CAB1_FCC1/35(REU_ELEV_mon_LOB_dat)
  SCP_elev_REU_LOB_mon/1(Out) -> [SCP_elev_REU_LOB_mon_1]
  [SCP_rud_REU1_LVDT_1] -> CAB1_FCC1/36(REU1_LVDT_rudder_dat)
  SCP_rud_REU1_LVDT/1(Out) -> [SCP_rud_REU1_LVDT_1]
  [SCP_rud_REU1_MON_1] -> CAB1_FCC1/37(REU1_MON_rudder_dat)
  SCP_rud_REU1_MON/1(Out) -> [SCP_rud_REU1_MON_1]
  [SCP_rud_REU2_LVDT_1] -> CAB1_FCC1/38(REU2_LVDT_rudder_dat)
  SCP_rud_REU2_LVDT/1(Out) -> [SCP_rud_REU2_LVDT_1]
  [SCP_rud_REU2_MON_1] -> CAB1_FCC1/39(REU2_MON_rudder_dat)
  SCP_rud_REU2_MON/1(Out) -> [SCP_rud_REU2_MON_1]
  [SCP_ail_REU_LOB_mon_1] -> CAB1_FCC1/40(REU_mon_AIL_LOB_dat)
  SCP_ail_REU_LOB_mon/1(Out) -> [SCP_ail_REU_LOB_mon_1]
  [SCP_elev_REU_RIB_LVDT_1] -> CAB1_FCC1/41(REU_ELEV_LVDT_RIB_dat)
  SCP_elev_REU_RIB_LVDT/1(Out) -> [SCP_elev_REU_RIB_LVDT_1]
  [SCP_elev_REU_RIB_mon_1] -> CAB1_FCC1/42(REU_ELEV_mon_RIB_dat)
  SCP_elev_REU_RIB_mon/1(Out) -> [SCP_elev_REU_RIB_mon_1]
  [SCP_elev_REU_ROB_LVDT_1] -> CAB1_FCC1/43(REU_ELEV_LVDT_ROB_dat)
  SCP_elev_REU_ROB_LVDT/1(Out) -> [SCP_elev_REU_ROB_LVDT_1]
  [SCP_ail_REU_LIB_LVDT_1] -> CAB1_FCC1/7(REU_LVDT_AIL_LIB_dat)
  SCP_ail_REU_LIB_LVDT/1(Out) -> [SCP_ail_REU_LIB_LVDT_1]
  CAB4_GPM2_fcc_ram_mon_ELEV_ROB_dat/2 -> SCP_CAB1_GPM2_fcc_ram_mon_ELEV_ROB/2(In2)  "<CAB4_GPM2_fcc_ram_mon_ELEV_ROB_dat>"
  CAB4_GPM2_fcc_ram_mon_ELEV_ROB_dat/1 -> SCP_CAB1_GPM2_fcc_ram_mon_ELEV_ROB/1(In1)  "<CAB4_GPM2_fcc_ram_mon_ELEV_ROB_dat>"
  CAB4_GPM2_fcc_ram_mon_ELEV_RIB_dat/2 -> SCP_CAB1_GPM2_fcc_ram_mon_ELEV_RIB/2(In2)  "<CAB4_GPM2_fcc_ram_mon_ELEV_RIB_dat>"
  CAB4_GPM2_fcc_ram_mon_ELEV_RIB_dat/1 -> SCP_CAB1_GPM2_fcc_ram_mon_ELEV_RIB/1(In1)  "<CAB4_GPM2_fcc_ram_mon_ELEV_RIB_dat>"
  CAB4_GPM2_fcc_ram_mon_ELEV_LOB_dat/2 -> SCP_CAB1_GPM2_fcc_ram_mon_ELEV_LOB/2(In2)  "<CAB4_GPM2_fcc_ram_mon_ELEV_LOB_dat>"
  CAB4_GPM2_fcc_ram_mon_ELEV_LOB_dat/1 -> SCP_CAB1_GPM2_fcc_ram_mon_ELEV_LOB/1(In1)  "<CAB4_GPM2_fcc_ram_mon_ELEV_LOB_dat>"
  CAB4_GPM2_fcc_ram_mon_ELEV_LIB_dat/2 -> SCP_CAB1_GPM2_fcc_ram_mon_ELEV_LIB/2(In2)  "<CAB4_GPM2_fcc_ram_mon_ELEV_LIB_dat>"
  CAB4_GPM2_fcc_ram_mon_ELEV_LIB_dat/1 -> SCP_CAB1_GPM2_fcc_ram_mon_ELEV_LIB/1(In1)  "<CAB4_GPM2_fcc_ram_mon_ELEV_LIB_dat>"
  CAB4_GPM2_fcc_ram_mon_AIL_ROB_dat/2 -> SCP_CAB1_GPM2_fcc_ram_mon_AIL_ROB/2(In2)  "<CAB4_GPM2_fcc_ram_mon_AIL_ROB_dat>"
  CAB4_GPM2_fcc_ram_mon_AIL_ROB_dat/1 -> SCP_CAB1_GPM2_fcc_ram_mon_AIL_ROB/1(In1)  "<CAB4_GPM2_fcc_ram_mon_AIL_ROB_dat>"
  CAB4_GPM2_fcc_ram_mon_AIL_RIB_dat/2 -> SCP_CAB1_GPM2_fcc_ram_mon_AIL_RIB/2(In2)  "<CAB4_GPM2_fcc_ram_mon_AIL_RIB_dat>"
  CAB4_GPM2_fcc_ram_mon_AIL_RIB_dat/1 -> SCP_CAB1_GPM2_fcc_ram_mon_AIL_RIB/1(In1)  "<CAB4_GPM2_fcc_ram_mon_AIL_RIB_dat>"
  CAB4_GPM2_fcc_ram_mon_AIL_LOB_dat/2 -> SCP_CAB1_GPM2_fcc_ram_mon_AIL_LOB/2(In2)  "<CAB4_GPM2_fcc_ram_mon_AIL_LOB_dat>"
  CAB4_GPM2_fcc_ram_mon_AIL_LOB_dat/1 -> SCP_CAB1_GPM2_fcc_ram_mon_AIL_LOB/1(In1)  "<CAB4_GPM2_fcc_ram_mon_AIL_LOB_dat>"
  CAB4_GPM2_fcc_ram_mon_AIL_LIB_dat/2 -> SCP_CAB1_GPM2_fcc_ram_mon_AIL_LIB/2(In2)  "<CAB4_GPM2_fcc_ram_mon_AIL_LIB_dat>"
  CAB4_GPM2_fcc_ram_mon_AIL_LIB_dat/1 -> SCP_CAB1_GPM2_fcc_ram_mon_AIL_LIB/1(In1)  "<CAB4_GPM2_fcc_ram_mon_AIL_LIB_dat>"
  CAB1_GPM2_fcc_ram_mon_rudder_UPR_dat/2 -> SCP_CAB1_GPM2_fcc_ram_mon_rudder_UPR/2(In2)  "<CAB1_GPM2_fcc_ram_mon_rudder_UPR_dat>"
  CAB1_GPM2_fcc_ram_mon_rudder_UPR_dat/1 -> SCP_CAB1_GPM2_fcc_ram_mon_rudder_UPR/1(In1)  "<CAB1_GPM2_fcc_ram_mon_rudder_UPR_dat>"
  CAB4_GPM2_fcc_ram_mon_rudder_LWR_dat/2 -> SCP_CAB1_GPM2_fcc_ram_mon_rudder_LWR/2(In2)  "<CAB4_GPM2_fcc_ram_mon_rudder_LWR_dat>"
  CAB4_GPM2_fcc_ram_mon_rudder_LWR_dat/1 -> SCP_CAB1_GPM2_fcc_ram_mon_rudder_LWR/1(In1)  "<CAB4_GPM2_fcc_ram_mon_rudder_LWR_dat>"
  CAB4_GPM2_pitch_roll_cmd/2 -> SCP_CAB1_GPM2_pitch_roll/2(In2)  "<CAB4_GPM2_pitch_roll_cmd>"
  CAB4_GPM2_pitch_roll_cmd/1 -> SCP_CAB1_GPM2_pitch_roll/1(In1)  "<CAB4_GPM2_pitch_roll_cmd>"
  CAB4_GPM2_yaw_cmd/2 -> SCP_CAB1_GPM2_yaw/2(In2)  "<CAB4_GPM2_yaw_cmd>"
  CAB4_GPM2_yaw_cmd/1 -> SCP_CAB1_GPM2_yaw/1(In1)  "<CAB4_GPM2_yaw_cmd>"
  elev_REU_RIB_LVDT_dat/2 -> SCP_elev_REU_RIB_LVDT/2(In2)  "<elev_REU_RIB_LVDT_dat>"
  elev_REU_ROB_mon_dat/2 -> SCP_elev_REU_ROB_mon/2(In2)  "<elev_REU_ROB_mon_dat>"
  elev_REU_ROB_mon_dat/1 -> SCP_elev_REU_ROB_mon/1(In1)  "<elev_REU_ROB_mon_dat>"
  elev_REU_ROB_LVDT_dat/2 -> SCP_elev_REU_ROB_LVDT/2(In2)  "<elev_REU_ROB_LVDT_dat>"
  elev_REU_ROB_LVDT_dat/1 -> SCP_elev_REU_ROB_LVDT/1(In1)  "<elev_REU_ROB_LVDT_dat>"
  elev_REU_RIB_mon_dat/2 -> SCP_elev_REU_RIB_mon/2(In2)  "<elev_REU_RIB_mon_dat>"
  elev_REU_RIB_mon_dat/1 -> SCP_elev_REU_RIB_mon/1(In1)  "<elev_REU_RIB_mon_dat>"
  elev_REU_RIB_LVDT_dat/1 -> SCP_elev_REU_RIB_LVDT/1(In1)  "<elev_REU_RIB_LVDT_dat>"
  rud_REU2_MON_dat/2 -> SCP_rud_REU2_MON/2(In2)  "<rud_REU2_MON_dat>"
  rud_REU2_MON_dat/1 -> SCP_rud_REU2_MON/1(In1)  "<rud_REU2_MON_dat>"
  rud_REU2_LVDT_dat/2 -> SCP_rud_REU2_LVDT/2(In2)  "<rud_REU2_LVDT_dat>"
  rud_REU2_LVDT_dat/1 -> SCP_rud_REU2_LVDT/1(In1)  "<rud_REU2_LVDT_dat>"
  rud_REU1_MON_dat/2 -> SCP_rud_REU1_MON/2(In2)  "<rud_REU1_MON_dat>"
  rud_REU1_MON_dat/1 -> SCP_rud_REU1_MON/1(In1)  "<rud_REU1_MON_dat>"
  rud_REU1_LVDT_dat/2 -> SCP_rud_REU1_LVDT/2(In2)  "<rud_REU1_LVDT_dat>"
  rud_REU1_LVDT_dat/1 -> SCP_rud_REU1_LVDT/1(In1)  "<rud_REU1_LVDT_dat>"
  elev_REU_LOB_mon_dat/2 -> SCP_elev_REU_LOB_mon/2(In2)  "<elev_REU_LOB_mon_dat>"
  elev_REU_LOB_mon_dat/1 -> SCP_elev_REU_LOB_mon/1(In1)  "<elev_REU_LOB_mon_dat>"
  elev_REU_LOB_LVDT_dat/2 -> SCP_elev_REU_LOB_LVDT/2(In2)  "<elev_REU_LOB_LVDT_dat>"
  elev_REU_LOB_LVDT_dat/1 -> SCP_elev_REU_LOB_LVDT/1(In1)  "<elev_REU_LOB_LVDT_dat>"
  elev_REU_LIB_mon_dat/2 -> SCP_elev_REU_LIB_mon_dat/2(In2)  "<elev_REU_LIB_mon_dat>"
  elev_REU_LIB_mon_dat/1 -> SCP_elev_REU_LIB_mon_dat/1(In1)  "<elev_REU_LIB_mon_dat>"
  elev_REU_LIB_LVDT_dat/2 -> SCP_elev_REU_LIB_LVDT/2(In2)  "<elev_REU_LIB_LVDT_dat>"
  elev_REU_LIB_LVDT_dat/1 -> SCP_elev_REU_LIB_LVDT/1(In1)  "<elev_REU_LIB_LVDT_dat>"
  ail_REU_ROB_mon_dat/2 -> SCP_ail_REU_ROB_mon/2(In2)  "<ail_REU_ROB_mon_dat>"
  ail_REU_ROB_mon_dat/1 -> SCP_ail_REU_ROB_mon/1(In1)  "<ail_REU_ROB_mon_dat>"
  ail_REU_ROB_LVDT_dat/2 -> SCP_ail_REU_ROB_LVDT/2(In2)  "<ail_REU_ROB_LVDT_dat>"
  ail_REU_ROB_LVDT_dat/1 -> SCP_ail_REU_ROB_LVDT/1(In1)  "<ail_REU_ROB_LVDT_dat>"
  ail_REU_RIB_mon_dat/2 -> SCP_ail_REU_RIB_mon/2(In2)  "<ail_REU_RIB_mon_dat>"
  ail_REU_RIB_mon_dat/1 -> SCP_ail_REU_RIB_mon/1(In1)  "<ail_REU_RIB_mon_dat>"
  ail_REU_RIB_LVDT_dat/2 -> SCP_ail_REU_RIB_LVDT/2(In2)  "<ail_REU_RIB_LVDT_dat>"
  ail_REU_RIB_LVDT_dat/1 -> SCP_ail_REU_RIB_LVDT/1(In1)  "<ail_REU_RIB_LVDT_dat>"
  ail_REU_LOB_mon_dat/2 -> SCP_ail_REU_LOB_mon/2(In2)  "<ail_REU_LOB_mon_dat>"
  ail_REU_LOB_mon_dat/1 -> SCP_ail_REU_LOB_mon/1(In1)  "<ail_REU_LOB_mon_dat>"
  ail_REU_LOB_LVDT_dat/2 -> SCP_ail_REU_LOB_LVDT/2(In2)  "<ail_REU_LOB_LVDT_dat>"
  ail_REU_LOB_LVDT_dat/1 -> SCP_ail_REU_LOB_LVDT/1(In1)  "<ail_REU_LOB_LVDT_dat>"
  ail_REU_LIB_mon_dat/2 -> SCP_ail_REU_LIB_mon/2(In2)  "<ail_REU_LIB_mon_dat>"
  ail_REU_LIB_mon_dat/1 -> SCP_ail_REU_LIB_mon/1(In1)  "<ail_REU_LIB_mon_dat>"
  ail_REU_LIB_LVDT_dat/2 -> SCP_ail_REU_LIB_LVDT/2(In2)  "<ail_REU_LIB_LVDT_dat>"
  ail_REU_LIB_LVDT_dat/1 -> SCP_ail_REU_LIB_LVDT/1(In1)  "<ail_REU_LIB_LVDT_dat>"
  [CAB1_FCC1_12_fcc_ram_mon_ELEV_ROB_dat] -> Outputs/12
  CAB1_FCC1/12(fcc_ram_mon_ELEV_ROB_dat) -> [CAB1_FCC1_12_fcc_ram_mon_ELEV_ROB_dat]  "fcc_ram_mon_ELEV_ROB_dat"
  [CAB1_FCC1_11_fcc_ram_mon_ELEV_RIB_dat] -> Outputs/11
  CAB1_FCC1/11(fcc_ram_mon_ELEV_RIB_dat) -> [CAB1_FCC1_11_fcc_ram_mon_ELEV_RIB_dat]  "fcc_ram_mon_ELEV_RIB_dat"